simulink model "MX_Gateway"  (Simulink 9.0) (continued)
  CD_FAST_TRAVEL_THROTTLE_LIMIT = Bobcat.Parameter; CreateAliasCD( CD_FAST_TRAVEL_THROTTLE_LIMIT                        ,uint16(1000)               ,'ConstantDataPointer' ,'CONSTANT_DATA' ,'FAST_TRAVEL_THROTTLE_LIMIT')    [cdata.m]    (also in hc12_cdata.m; m4_cdata.m)
  CD_FLYWHEEL_TEETH = Bobcat.Parameter; CreateAliasCD( CD_FLYWHEEL_TEETH                                    ,uint8(83)                  ,'ConstantDataPointer' ,'CONSTANT_DATA' ,'FLYWHEEL_TEETH')    [cdata.m]    (also in hc12_cdata.m; m4_cdata.m)
  CD_FUEL_LEVEL_FILTER_FAST_VALUE = Bobcat.Parameter; CreateAliasCD( CD_FUEL_LEVEL_FILTER_FAST_VALUE                      ,uint8(5)                   ,'ConstantDataPointer' ,'CONSTANT_DATA' ,'FUEL_LEVEL_FILTER_FAST_VALUE')    [cdata.m]    (also in hc12_cdata.m; m4_cdata.m)
  CD_FUEL_LEVEL_FILTER_SLOW_VALUE = Bobcat.Parameter; CreateAliasCD( CD_FUEL_LEVEL_FILTER_SLOW_VALUE                      ,uint8(10)                  ,'ConstantDataPointer' ,'CONSTANT_DATA' ,'FUEL_LEVEL_FILTER_SLOW_VALUE')    [cdata.m]    (also in hc12_cdata.m; m4_cdata.m)
  CD_FUEL_PRIMARY_FEEDBACK_FUNCTION = Bobcat.Parameter; CreateAliasCD( CD_FUEL_PRIMARY_FEEDBACK_FUNCTION                    ,uint8(0)                   ,'ConstantDataPointer' ,'CONSTANT_DATA' ,'FUEL_PRIMARY_FEEDBACK_FUNCTION')    [cdata.m]    (also in hc12_cdata.m; m4_cdata.m)
  ... (+246 more)
undefined symbols (not defined in the file or in its folder): sigbuilder_block
undefined symbols (not defined in the file): CD_FUEL_RESOLUTION_TIMER
libraries: simulink x186, MX_Library x107
(part 10 of 48)

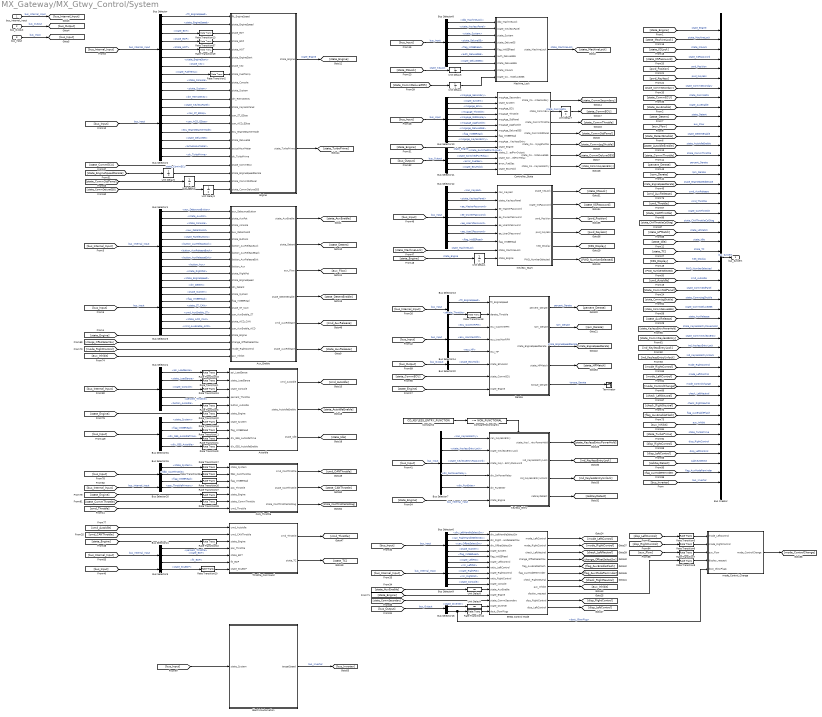
== system MX_Gateway/MX_Gtwy_Control/System ==
  bus_Internal_Input : Inport
  bus_Output : Inport  Port=2
  bus_Input : Inport  Port=3
  AutoIdle : Subsystem  RequirementInfo={} %GIDa_b4de8e37_b570_4228_8969_fb5e451b31f8  TreatAsAtomicUnit=on  SystemSampleTime=srate100  RTWFcnName=mod_engine_control  RTWFileName=mod_System
      settings: RTWSystemCode=Nonreusable function  RTWFileNameOpts=User specified
  Aux_Enable : Subsystem  TreatAsAtomicUnit=on  SystemSampleTime=srate  RTWFileName=mod_System
      settings: RTWSystemCode=Nonreusable function  RTWFcnNameOpts=Use subsystem name  RTWFileNameOpts=User specified
  Bus Creator : BusCreator  Inputs=48
  Bus Selector : BusSelector  OutputSignals=flt_EngineSpeed,state_EngineSpeed,state_ECT,state_EOP,state_HOT,state_EngineStart,state_Key,state_FuelTemp,state_Console
  Bus Selector1 : BusSelector  OutputSignals=aux_DebounceButton,state_AuxPot,state_Console,aux_DetentHold,state_PanelButtons,button_AuxReleaseAck,button_AuxReleaseBack,button_AuxReleaseExit,button_Aux,state_RightPot,state_EngineSpeed
  Bus Selector10 : BusSelector  OutputSignals=bus_CAN.bus_Secondary.msgAge_Secondary,bus_Controller.state_System,bus_CAN.bus_ECU.msgAge_ECU,bus_CAN.bus_Throttle.msgAge_Throttle,bus_CAN.bus_StdDisplay.msgAge_StdDisplay,bus_CAN.bus_JogShuttle.msgAge_JogShuttle,bus_CAN.bus_DeluxeG55.msgAge_DeluxeG55,bus_Controller.flag_InitEERead,bus_CAN.bus_KeylessEntry.msgAge_KeylessEntry
  Bus Selector11 : BusSelector  OutputSignals=state_SwitchedPwrOutput,state_SwitchedPwrRelay,error_FuelSec,state_ECUHold
  Bus Selector12 : BusSelector  OutputSignals=bus_CAN.bus_Keypad.can_Keypad,bus_CAN.bus_Keypad.state_KeylessPanel,bus_Controller.ee_MasterPassword,bus_Controller.ee_OwnerPassword,bus_Controller.ee_User1Password,bus_Controller.ee_User2Password,bus_Controller.flag_InitEERead
  Bus Selector13 : BusSelector  OutputSignals=flt_EngineSpeed,derate_Throttle
  Bus Selector14 : BusSelector  OutputSignals=bus_CAN.bus_ECU.ecu_isocMinRPM,bus_CAN.bus_ECU.ecu_isocMaxRPM,bus_CAN.bus_ECU.ecu_HP
  Bus Selector15 : BusSelector  OutputSignals=bus_Controller.state_System,bus_CAN.bus_Throttle.fdbk_CANThrottle,bus_Controller.flag_InitEERead
  Bus Selector16 : BusSelector  OutputSignals=state_Diverter,dout_GlowPlug
  Bus Selector2 : BusSelector  OutputSignals=bus_Controller.state_System,bus_DigitalInputs.din_RemoteKey,bus_CAN.bus_Keypad.state_KeylessPanel,bus_CAN.bus_ServiceTool.can_ST_EStop,bus_CAN.bus_ACD.can_ACD_EStop,bus_CAN.bus_ECU.ecu_EngineStarterMode,bus_CAN.bus_DeluxeG5.state_DeluxeG5,bus_Controller.actualHourMeter,bus_Controller.dv_TurboPrime
  Bus Selector20 : BusSelector  OutputSignals=pos_ThrottlePrimary
  Bus Selector21 : BusSelector  OutputSignals=percent_Throttle,state_EOT,flt_EOP
  Bus Selector22 : BusSelector  OutputSignals=state_ECUHold
  Bus Selector3 : BusSelector  OutputSignals=bus_DigitalInputs.din_Detent,bus_Controller.state_System,bus_Controller.flag_InitEERead,bus_CAN.bus_ServiceTool.state_ST_CAN,bus_CAN.bus_ServiceTool.cmd_AuxEnable_ST,bus_CAN.bus_ACD.state_ACD_CAN,bus_CAN.bus_ACD.cmd_AuxEnable_ACD
  Bus Selector31 : BusSelector  OutputSignals=bus_Controller.state_System,bus_Controller.flag_InitEERead,bus_CAN.bus_DeluxeG55.dlx_G55_AutoIdleTime,bus_CAN.bus_DeluxeG55.dlx_G55_AutoIdle
  Bus Selector4 : BusSelector  OutputSignals=psi_LoadSense,state_LoadSense,state_Console,percent_Throttle,button_AutoIdle
  Bus Selector5 : BusSelector  OutputSignals=bus_CAN.bus_ECU.state_ecuEOP
  Bus Selector6 : BusSelector  OutputSignals=bus_DigitalInputs.din_LeftHandleSelectSw,bus_CAN.bus_Secondary.can_RightHandleSelectSw,bus_CAN.bus_Secondary.can_OffsetSelectSw,bus_Controller.state_System,bus_Controller.flag_InitEERead
  Bus Selector7 : BusSelector  OutputSignals=bus_CAN.bus_KeylessEntry.can_KeylessEntry,bus_Controller.state_KeylessEntryLock,bus_Controller.state_KeylessEntryPassword,bus_DigitalInputs.din_SwPowerRelay,bus_DigitalInputs.din_RunEnter
  Bus Selector8 : BusSelector  OutputSignals=bus_Controller.ddp_MachineLock,bus_CAN.bus_Keypad.state_KeylessPanel,bus_Controller.state_System,bus_CAN.bus_DeluxeG5.state_DeluxeG5,bus_Controller.flag_InitEERead,bus_CAN.bus_DeluxeG55.auth_DeluxeG55,bus_CAN.bus_DeluxeG55.state_DeluxeG55
  Bus Selector9 : BusSelector  OutputSignals=state_LeftPot,rm_LeftPot,state_RightPot,rm_RightPot,state_Console
  CAN_Throttle : Subsystem  TreatAsAtomicUnit=on  SystemSampleTime=srate100  RTWFileName=mod_System
      settings: RTWSystemCode=Nonreusable function  RTWFileNameOpts=User specified
  CompareToConstant1 : simulink/Logic and Bit Operations/Compare To Constant  relop=~=  const=NON_FUNCTIONAL  OutDataTypeStr=boolean  ZeroCross=off
  Constant2 : Constant  Value=CD_KEYLESS_ENTRY_FUNCTION
  Controller_State : Subsystem  TreatAsAtomicUnit=on  SystemSampleTime=srate  RTWFileName=mod_System
      settings: RTWSystemCode=Nonreusable function  RTWFileNameOpts=User specified
  Derate : Subsystem  TreatAsAtomicUnit=on  SystemSampleTime=srate  RTWFileName=mod_System
      settings: RTWSystemCode=Nonreusable function  RTWFileNameOpts=User specified
  EMEA Control Mode : Subsystem  TreatAsAtomicUnit=on  RTWFileName=mod_System
      settings: RTWSystemCode=Nonreusable function  RTWFileNameOpts=User specified
  ElectricAutomation : Subsystem  TreatAsAtomicUnit=on  SystemSampleTime=srate100  RTWFcnName=mod_engine_control  RTWFileName=mod_System
      settings: RTWSystemCode=Nonreusable function  RTWFileNameOpts=User specified
  Engine : Subsystem  TreatAsAtomicUnit=on  SystemSampleTime=srate  RTWFileName=mod_System
      settings: RTWSystemCode=Nonreusable function  RTWFcnNameOpts=Use subsystem name  RTWFileNameOpts=User specified
  Keyless_Entry : Subsystem  TreatAsAtomicUnit=on  SystemSampleTime=srate  RTWFileName=mod_System
      settings: RTWSystemCode=Nonreusable function  RTWFileNameOpts=User specified
  Keyless_Start : Subsystem  TreatAsAtomicUnit=on  SystemSampleTime=srate  RTWFileName=mod_System
      settings: RTWSystemCode=Nonreusable function  RTWFileNameOpts=User specified
  Machine_Lock : Subsystem  TreatAsAtomicUnit=on  SystemSampleTime=srate  RTWFileName=mod_System
      settings: RTWSystemCode=Nonreusable function  RTWFileNameOpts=User specified
  Rate Transition{1..4,9..13,17..20,22..28,57,64} (22 alike) : RateTransition  Integrity=off
  Rate Transition5 : RateTransition  Integrity=off  OutPortSampleTime=srate100
  Rate Transition6 : RateTransition  Integrity=off  OutPortSampleTime=srate100
  Rate Transition7 : RateTransition  Integrity=off  OutPortSampleTime=srate100
  Rate Transition8 : RateTransition  OutPortSampleTime=srate100
  Terminator : Terminator
  Throttle_Command : Subsystem  TreatAsAtomicUnit=on  RTWFileName=mod_System
      settings: RTWSystemCode=Nonreusable function  RTWFileNameOpts=User specified
  Unit Delay{1..9} (9 alike) : UnitDelay  SampleTime=-1
      settings: AttributesFormatString=<tsample=%<SampleTime>>\n<initial=%<X0>>
  mode_Control_Change : Subsystem
  bus_System : Outport
  wires:
  [state_Engine] -> Bus Creator/1  "state_Engine"
  Bus Selector/4 -> Rate Transition57/1  "<state_EOP>"
  Rate Transition57/1 -> Engine/4(state_EOP)
  Bus Selector/2 -> Engine/2(state_EngineSpeed)  "<state_EngineSpeed>"
  Engine/1(state_Engine) -> [state_Engine]  "state_Engine"
  Bus Selector/3 -> Rate Transition13/1  "<state_ECT>"
  Bus Selector/6 -> Engine/6(state_EngineStart)  "<state_EngineStart>"
  Bus Selector/7 -> Engine/7(state_Key)  "<state_Key>"
  Rate Transition13/1 -> Engine/3(state_ECT)
  Rate Transition19/1 -> Engine/5(state_HOT)
  bus_Internal_Input/1 -> [bus_Internal_Input]  "bus_Internal_Input"
  bus_Output/1 -> [bus_Output]  "bus_Output"
  bus_Input/1 -> [bus_Input]  "bus_Input"
  [bus_Internal_Input] -> Bus Selector/1  "bus_Internal_Input"
  [bus_Input] -> Bus Selector2/1  "bus_Input"
  Bus Selector/1 -> Engine/1(flt_EngineSpeed)  "<flt_EngineSpeed>"
  Bus Selector/5 -> Rate Transition19/1  "<state_HOT>"
  [bus_Input] -> Bus Selector8/1  "bus_Input"
  Bus Selector8/1 -> Machine_Lock/1(ddp_MachineLock)  "<ddp_MachineLock>"
  Machine_Lock/1(state_MachineLock) -> [state_MachineLock]  "state_MachineLock"
  [state_MachineLock] -> Bus Creator/2  "state_MachineLock"
  Bus Selector8/2 -> Machine_Lock/2(state_KeylessPanel)  "<state_KeylessPanel>"
  [state_KSLock] -> Bus Creator/3  "state_KSLock"
  [state_KSPassword] -> Bus Creator/4  "state_KSPassword"
  [pwd_Position] -> Bus Creator/5  "pwd_Position"
  [pwd_Keyless] -> Bus Creator/6  "pwd_Keyless"
  Bus Selector8/3 -> Machine_Lock/3(state_System)  "<state_System>"
  [state_KSLock] -> Unit Delay5/1  "state_KSLock"
  Unit Delay5/1 -> Machine_Lock/8(state_KSLock)
  Bus Selector8/4 -> Machine_Lock/4(state_DeluxeG5)  "<state_DeluxeG5>"
  Bus Selector8/5 -> Machine_Lock/5(flag_InitEERead)  "<flag_InitEERead>"
  Controller_State/1(state_CommSecondary) -> [state_CommSecondary]
  [bus_Input] -> Bus Selector10/1  "bus_Input"
  Bus Selector10/1 -> Controller_State/1(msgAge_Secondary)  "<msgAge_Secondary>"
  Bus Selector10/2 -> Controller_State/2(state_System)  "<state_System>"
  [state_Engine] -> Controller_State/10(state_Engine)  "state_Engine"
  [bus_Output] -> Bus Selector11/1  "bus_Output"
  Bus Selector11/2 -> Controller_State/12(state_SwitchedPwrRelay)  "<state_SwitchedPwrRelay>"
  [state_CommSecondary] -> Bus Creator/7  "state_CommSecondary"
  Bus Selector10/3 -> Controller_State/3(msgAge_ECU)  "<msgAge_ECU>"
  Controller_State/2(state_CommECU) -> Unit Delay7/1  "state_CommECU"
  [state_CommECU] -> Bus Creator/8  "state_CommECU"
  [state_CommECU] -> Engine/19(state_CommECU)  "state_CommECU"
  Unit Delay7/1 -> [state_CommECU]
  Bus Selector11/1 -> Controller_State/11(state_SwitchedPwrOutput)  "<state_SwitchedPwrOutput>"
  Bus Selector11/3 -> Controller_State/13(error_FuelSec)  "<error_FuelSec>"
  Bus Selector11/4 -> Controller_State/14(state_ECUHold)  "<state_ECUHold>"
  Bus Selector2/1 -> Engine/10(state_System)  "<state_System>"
  Bus Selector2/2 -> Engine/11(din_RemoteKey)  "<din_RemoteKey>"
  Bus Selector2/3 -> Engine/12(state_KeylessPanel)  "<state_KeylessPanel>"
  Bus Selector2/5 -> Engine/14(can_ACD_EStop)  "<can_ACD_EStop>"
  Bus Selector2/6 -> Engine/15(ecu_EngineStarterMode)  "<ecu_EngineStarterMode>"
  [state_AuxEnable] -> Bus Creator/9  "state_AuxEnable"
  Aux_Enable/1(state_AuxEnable) -> [state_AuxEnable]
  [bus_Internal_Input] -> Bus Selector1/1  "bus_Internal_Input"
  Bus Selector1/1 -> Aux_Enable/1(aux_DebounceButton)  "<aux_DebounceButton>"
  Bus Selector1/2 -> Aux_Enable/2(state_AuxPot)  "<state_AuxPot>"
  [state_Engine] -> Aux_Enable/19(state_Engine)
  [bus_Input] -> Bus Selector3/1  "bus_Input"
  Bus Selector3/1 -> Aux_Enable/12(din_Detent)  "<din_Detent>"
  Aux_Enable/2(state_Detent) -> [state_Detent]
  Aux_Enable/3(aux_Flow) -> [aux_Flow]
  Aux_Enable/4(state_DetentEnable) -> [state_DetentEnable]
  [state_Detent] -> Bus Creator/10  "state_Detent"
  [aux_Flow] -> Bus Creator/11  "aux_Flow"
  [state_DetentEnable] -> Bus Creator/12  "state_DetentEnable"
  [state_AutoIdleEnable] -> Bus Creator/13  "state_AutoIdleEnable"
  Bus Selector10/4 -> Controller_State/4(msgAge_Throttle)  "<msgAge_Throttle>"
  Controller_State/3(state_CommThrottle) -> [state_CommThrottle]
  [state_CommThrottle] -> Bus Creator/14  "state_CommThrottle"
  [percent_Derate] -> Bus Creator/15  "percent_Derate"
  [rpm_Derate] -> Bus Creator/16  "rpm_Derate"
  [state_EngineSpeedDerate] -> Bus Creator/17  "state_EngineSpeedDerate"
  Bus Selector1/3 -> Aux_Enable/3(state_console)  "<state_Console>"
  Bus Selector1/4 -> Aux_Enable/4(aux_DetentHold)  "<aux_DetentHold>"
  Bus Selector3/2 -> Aux_Enable/13(state_System)  "<state_System>"
  [state_EngineSpeedDerate] -> Unit Delay6/1
  Unit Delay6/1 -> Engine/20(state_EngineSpeedDerate)
  [bus_Internal_Input] -> Bus Selector13/1  "bus_Input"
  Bus Selector13/1 -> Derate/1(flt_EngineSpeed)  "<flt_EngineSpeed>"
  [bus_Input] -> Bus Selector14/1  "bus_Input"
  Derate/1(percent_Derate) -> [percent_Derate]  "percent_Derate"
  Derate/2(rpm_Derate) -> [rpm_Derate]  "rpm_Derate"
  Bus Selector14/1 -> Derate/3(ecu_isocMinRPM)  "<ecu_isocMinRPM>"
  Bus Selector14/2 -> Derate/4(ecu_isocMaxRPM)  "<ecu_isocMaxRPM>"
  Derate/3(state_EngineSpeedDerate) -> [state_EngineSpeedDerate]  "state_EngineSpeedDerate"
  Bus Selector13/2 -> Rate Transition18/1  "<derate_Throttle>"
  Rate Transition18/1 -> Derate/2(derate_Throttle)
  Bus Selector3/3 -> Aux_Enable/14(flag_InitEERead)  "<flag_InitEERead>"
  Bus Selector1/5 -> Aux_Enable/5(state_Buttons)  "<state_PanelButtons>"
  Bus Selector2/4 -> Engine/13(can_ST_EStop)  "<can_ST_EStop>"
  Bus Selector3/4 -> Aux_Enable/15(state_ST_CAN)  "<state_ST_CAN>"
  Bus Selector3/5 -> Aux_Enable/16(can_AuxEnable_ST)  "<cmd_AuxEnable_ST>"
  Bus Selector3/6 -> Aux_Enable/17(state_ACD_CAN)  "<state_ACD_CAN>"
  Bus Selector3/7 -> Aux_Enable/18(can_AuxEnable_ACD)  "<cmd_AuxEnable_ACD>"
  Aux_Enable/5(cmd_AuxRelease) -> [cmd_AuxRelease]
  [cmd_AuxRelease] -> Bus Creator/18  "cmd_AuxRelease"
  Bus Selector1/6 -> Aux_Enable/6(button_AuxReleaseAck)  "<button_AuxReleaseAck>"
  Bus Selector1/7 -> Aux_Enable/7(button_AuxReleaseBack)  "<button_AuxReleaseBack>"
  Bus Selector1/8 -> Aux_Enable/8(button_AuxReleaseExit)  "<button_AuxReleaseExit>"
  Rate Transition24/1 -> AutoIdle/3(state_Console)
  Rate Transition22/1 -> AutoIdle/6(state_Engine)
  Rate Transition23/1 -> AutoIdle/1(psi_LoadSense)
  [bus_Input] -> Bus Selector31/1
  Bus Selector31/1 -> Rate Transition64/1  "<state_System>"
  Rate Transition64/1 -> AutoIdle/7(state_System)
  [bus_Internal_Input] -> Bus Selector4/1
  Bus Selector4/1 -> Rate Transition23/1  "<psi_LoadSense>"
  Bus Selector4/3 -> Rate Transition24/1  "<state_Console>"
  Bus Selector4/4 -> AutoIdle/4(percent_Throttle)  "<percent_Throttle>"
  [state_Engine] -> Rate Transition22/1
  Bus Selector4/2 -> Rate Transition1/1  "<state_LoadSense>"
  Rate Transition1/1 -> AutoIdle/2(state_LoadSense)
  AutoIdle/1(cmd_AutoIdle) -> [cmd_AutoIdle]
  [cmd_Throttle] -> Bus Creator/19  "cmd_Throttle"
  AutoIdle/2(state_AutoIdleEnable) -> [state_AutoIdleEnable]
  [bus_Input] -> Bus Selector15/1
  Bus Selector15/1 -> Rate Transition17/1  "<state_System>"
  Bus Selector15/2 -> Rate Transition25/1  "<fdbk_CANThrottle>"
  Bus Selector15/3 -> Rate Transition26/1  "<flag_InitEERead>"
  [state_Engine] -> Rate Transition27/1
  [state_CommThrottle] -> Rate Transition28/1
  [bus_Internal_Input] -> Bus Selector20/1  "bus_Internal_Input"
  Bus Selector20/1 -> CAN_Throttle/4(pos_Throttle)  "<pos_ThrottlePrimary>"
  CAN_Throttle/1(cmd_CANThrottle) -> [cmd_CANThrottle]
  CAN_Throttle/2(state_CANThrottle) -> [state_CANThrottle]
  CAN_Throttle/3(state_CANThrottleCalDiag) -> [state_CANThrottleCalDiag]
  [state_CANThrottle] -> Bus Creator/20  "state_CANThrottle"
  [state_CANThrottleCalDiag] -> Bus Creator/21  "state_CANThrottleCalDiag"
  Rate Transition17/1 -> CAN_Throttle/1(state_System)
  Rate Transition25/1 -> CAN_Throttle/2(fdbk_CANThrottle)
  Rate Transition26/1 -> CAN_Throttle/3(flag_InitEERead)
  Rate Transition27/1 -> CAN_Throttle/5(state_Engine)
  Rate Transition28/1 -> CAN_Throttle/6(state_CommThrottle)
  Bus Creator/1 -> bus_System/1  "bus_System"
  [state_HPMatch] -> Bus Creator/22  "state_HPMatch"
  Derate/4(state_HPMatch) -> [state_HPMatch]
  [bus_Output] -> Bus Selector22/1  "bus_Output"
  Bus Selector14/3 -> Derate/5(ecu_HP)  "<ecu_HP>"
  Bus Selector22/1 -> Derate/6(state_ECUHold)  "<state_ECUHold>"
  [state_CommECU] -> Derate/7(state_CommECU)
  Bus Selector/8 -> Rate Transition2/1  "<state_FuelTemp>"
  AutoIdle/3(state_Idle) -> [state_Idle]
  [state_Idle] -> Bus Creator/23  "state_Idle"
  Rate Transition2/1 -> Engine/8(state_FuelTemp)
  Throttle_Command/1(cmd_Throttle) -> [cmd_Throttle]
  [cmd_AutoIdle] -> Throttle_Command/1(cmd_AutoIdle)
  [bus_Internal_Input] -> Bus Selector21/1  "bus_Internal_Input"
  Bus Selector21/1 -> Throttle_Command/4(pos_Throttle)  "<percent_Throttle>"
  [cmd_CANThrottle] -> Throttle_Command/2(cmd_CANThrottle)
  Throttle_Command/2(state_TC) -> [state_TC]
  Bus Selector21/2 -> Throttle_Command/5(state_EOT)  "<state_EOT>"
  [state_TC] -> Bus Creator/24  "state_TC"
  [state_Engine] -> Rate Transition10/1
  Bus Selector4/5 -> Rate Transition3/1  "<button_AutoIdle>"
  Rate Transition3/1 -> AutoIdle/5(button_AutoIdle)
  Rate Transition10/1 -> Throttle_Command/3(state_Engine)
  [cmd_Throttle] -> CAN_Throttle/7(cmd_Throttle)
  [bus_Input] -> Bus Selector5/1
  Bus Selector5/1 -> Rate Transition20/1  "<state_ecuEOP>"
  Bus Selector21/3 -> Throttle_Command/6(flt_EOP)  "<flt_EOP>"
  Rate Transition20/1 -> Throttle_Command/7(state_ecuEOP)
  Bus Selector1/9 -> Aux_Enable/9(button_Aux)  "<button_Aux>"
  Keyless_Start/4(pwd_Keyless) -> [pwd_Keyless]
  Keyless_Start/3(pwd_Position) -> [pwd_Position]
  Keyless_Start/2(state_KSPassword) -> [state_KSPassword]
  Keyless_Start/1(state_KSLock) -> [state_KSLock]
  [bus_Input] -> Bus Selector12/1  "bus_Input"
  Keyless_Start/5(KSS_Display) -> [KSS_Display]
  Keyless_Start/6(PWD_NumberSelected) -> [PWD_NumberSelected]
  Bus Selector12/1 -> Keyless_Start/1(can_Keypad)  "<can_Keypad>"
  Bus Selector12/2 -> Keyless_Start/2(state_KeylessPanel)  "<state_KeylessPanel>"
  Bus Selector12/5 -> Keyless_Start/5(ee_User1Password)  "<ee_User1Password>"
  Bus Selector12/6 -> Keyless_Start/6(ee_User2Password)  "<ee_User2Password>"
  [state_MachineLock] -> Keyless_Start/8(state_MachineLock)  "state_MachineLock"
  [state_Engine] -> Unit Delay1/1  "state_Engine"
  Unit Delay1/1 -> Keyless_Start/9(state_Engine)
  [KSS_Display] -> Bus Creator/25  "KSS_Display"
  [PWD_NumberSelected] -> Bus Creator/26  "PWD_NumberSelected"
  Bus Selector12/3 -> Keyless_Start/3(ee_MasterPassword)  "<ee_MasterPassword>"
  Bus Selector12/4 -> Keyless_Start/4(ee_OwnerPassword)  "<ee_OwnerPassword>"
  Rate Transition11/1 -> AutoIdle/8(flag_InitEERead)
  Bus Selector31/2 -> Rate Transition11/1  "<flag_InitEERead>"
  Bus Selector12/7 -> Keyless_Start/7(flag_InitEERead)  "<flag_InitEERead>"
  [state_Engine] -> Derate/8(state_Engine)
  Derate/5(torque_Derate) -> Terminator/1  "torque_Derate"
  [cmd_AutoIdle] -> Bus Creator/27  "cmd_AutoIdle"
  Bus Selector10/7 -> Controller_State/7(msgAge_DeluxeG55)  "<msgAge_DeluxeG55>"
  Bus Selector10/5 -> Controller_State/5(msgAge_StdPanel)  "<msgAge_StdDisplay>"
  Bus Selector10/6 -> Controller_State/6(msgAge_JogShuttle)  "<msgAge_JogShuttle>"
  Controller_State/4(state_CommStdPanel) -> [state_CommStdPanel]
  Controller_State/5(state_CommJogShuttle) -> [state_CommJogShuttle]
  Controller_State/6(state_CommDeluxeG55) -> [state_CommDeluxeG55]
  [state_CommDeluxeG55] -> Bus Creator/30  "state_CommDeluxeG55"
  [state_CommStdPanel] -> Bus Creator/28  "state_CommStdPanel"
  [state_CommJogShuttle] -> Bus Creator/29  "state_CommJogShuttle"
  Bus Selector2/7 -> Engine/16(state_DeluxeG5)  "<state_DeluxeG5>"
  [state_CommDeluxeG55] -> Unit Delay3/1
  [state_CommStdPanel] -> Unit Delay2/1
  Unit Delay2/1 -> Engine/21(state_CommStdPanel)
  Unit Delay3/1 -> Engine/22(state_CommDeluxeG55)
  Rate Transition4/1 -> AutoIdle/9(dlx_G55_AutoIdleTime)
  Bus Selector31/3 -> Rate Transition4/1  "<dlx_G55_AutoIdleTime>"
  Aux_Enable/6(state_AuxRelease) -> [state_AuxRelease]
  [state_AuxRelease] -> Bus Creator/31  "state_AuxRelease"
  Bus Selector10/8 -> Controller_State/8(flag_InitEERead)  "<flag_InitEERead>"
  Bus Selector/9 -> Engine/9(state_Console)  "<state_Console>"
  Bus Selector8/6 -> Machine_Lock/6(auth_DeluxeG55)  "<auth_DeluxeG55>"
  [state_CommDeluxeG55] -> Unit Delay4/1
  Unit Delay4/1 -> Machine_Lock/9(state_CommDeluxeG55)
  [bus_Input] -> Bus Selector7/1  "bus_Input"
  Bus Selector7/1 -> Keyless_Entry/1(can_KeylessEntry)  "<can_KeylessEntry>"
  Keyless_Entry/1(state_KeylessEntryPowerHold) -> [state_KeylessEntryPowerHold]
  [state_KeylessEntryPowerHold] -> Bus Creator/32  "state_KeylessEntryPowerHold"
  Bus Selector10/9 -> Controller_State/9(msgAge_KeylessEntry)  "<msgAge_KeylessEntry>"
  Controller_State/7(state_CommKeylessEntry) -> [state_CommKeylessEntry]
  [state_CommKeylessEntry] -> Bus Creator/33  "state_CommKeylessEntry"
  Constant2/1 -> CompareToConstant1/1
  CompareToConstant1/1 -> Keyless_Entry/Enable
  Keyless_Entry/2(ind_KeylessEntryLock) -> [ind_KeylessEntryLock]
  Keyless_Entry/3(ind_KeylessEntryUnlock) -> [ind_KeylessEntryUnlock]
  [ind_KeylessEntryLock] -> Bus Creator/34  "ind_KeylessEntryLock"
  [ind_KeylessEntryUnlock] -> Bus Creator/35  "ind_KeylessEntryUnlock"
  Bus Selector2/8 -> Engine/17(actualHourMeter)  "<actualHourMeter>"
  Bus Selector2/9 -> Engine/18(dv_TurboPrime)  "<dv_TurboPrime>"
  [bus_Input] -> Bus Selector6/1  "bus_Input"
  Bus Selector6/1 -> EMEA Control Mode/1(din_LeftHandleSelectSw)  "<din_LeftHandleSelectSw>"
  [bus_Internal_Input] -> Bus Selector9/1  "bus_Internal_Input"
  Bus Selector9/1 -> EMEA Control Mode/6(state_LeftControl)  "<state_LeftPot>"
  Bus Selector9/2 -> EMEA Control Mode/7(ratio_LeftControl)  "<rm_LeftPot>"
  Bus Selector9/3 -> EMEA Control Mode/8(state_RightControl)  "<state_RightPot>"
  Bus Selector9/4 -> EMEA Control Mode/9(ratio_RightControl)  "<rm_RightPot>"
  [state_AuxEnable] -> Unit Delay8/1
  [mode_RightControl] -> Bus Creator/36  "mode_RightControl"
  [mode_LeftControl] -> Bus Creator/37  "mode_LeftControl"
  [mode_ControlChange] -> Bus Creator/38  "mode_ControlChange"
  Bus Selector6/2 -> EMEA Control Mode/2(din_RightHandleSelectSw)  "<can_RightHandleSelectSw>"
  Bus Selector6/3 -> EMEA Control Mode/3(din_OffsetSelectSw)  "<can_OffsetSelectSw>"
  EMEA Control Mode/1(mode_LeftControl) -> [mode_LeftControl]
  EMEA Control Mode/2(mode_RightControl) -> [mode_RightControl]
  Bus Selector1/10 -> Aux_Enable/10(state_RightPot)  "<state_RightPot>"
  EMEA Control Mode/3(check_LeftNeutral) -> [check_LeftNeutral]
  [check_LeftNeutral] -> Bus Creator/39  "check_LeftNeutral"
  [aux_Flow] -> Rate Transition7/1
  EMEA Control Mode/4(change_OffsetSelectSw) -> [change_OffsetSelectSw]
  [change_OffsetSelectSw] -> Aux_Enable/20(change_OffsetSelectSw)
  [mode_RightControl] -> Aux_Enable/21(mode_RightControl)
  Bus Selector6/4 -> EMEA Control Mode/4(state_System)  "<state_System>"
  Bus Selector9/5 -> EMEA Control Mode/10(state_console)  "<state_Console>"
  [state_Engine] -> EMEA Control Mode/12(state_Engine)
  Unit Delay8/1 -> EMEA Control Mode/11(state_AuxEnable)
  [check_RightNeutral] -> Bus Creator/40  "check_RightNeutral"
  [flag_AuxEnableFlash] -> Bus Creator/41  "flag_AuxEnableFlash"
  EMEA Control Mode/5(flag_AuxEnableFlash) -> [flag_AuxEnableFlash]
  EMEA Control Mode/7(check_RightNeutral) -> [check_RightNeutral]
  EMEA Control Mode/8(aux_Inhibit) -> [aux_Inhibit]
  [aux_Inhibit] -> Aux_Enable/22(aux_Inhibit)
  [aux_Inhibit] -> Bus Creator/42  "aux_Inhibit"
  [state_CommSecondary] -> EMEA Control Mode/13(state_CommSecondary)
  [bus_Output] -> Bus Selector16/1  "bus_Output"
  Bus Selector16/1 -> Unit Delay9/1  "<state_Diverter>"
  Unit Delay9/1 -> EMEA Control Mode/14(state_Diverter)
  Rate Transition5/1 -> mode_Control_Change/1(mode_LeftControl)
  Rate Transition6/1 -> mode_Control_Change/2(mode_RightControl)
  Rate Transition7/1 -> mode_Control_Change/3(aux_Flow)
  mode_Control_Change/1(mode_ControlChange) -> [mode_ControlChange]
  EMEA Control Mode/9(display_request) -> Rate Transition8/1
  Rate Transition8/1 -> mode_Control_Change/4(display_request)
  Engine/2(state_TurboPrime) -> [state_TurboPrime]
  [state_TurboPrime] -> Bus Creator/43  "state_TurboPrime"
  Bus Selector8/7 -> Machine_Lock/7(state_DeluxeG55)  "<state_DeluxeG55>"
  Bus Selector1/11 -> Aux_Enable/11(state_EngineSpeed)  "<state_EngineSpeed>"
  Bus Selector6/5 -> EMEA Control Mode/5(flag_InitEERead)  "<flag_InitEERead>"
  Bus Selector7/2 -> Keyless_Entry/2(state_KeylessEntryLock)  "<state_KeylessEntryLock>"
  Bus Selector7/3 -> Keyless_Entry/3(state_KeylessEntryPassword)  "<state_KeylessEntryPassword>"
  EMEA Control Mode/11(disp_LeftControl) -> [disp_LeftControl]
  EMEA Control Mode/10(disp_RightControl) -> [disp_RightControl]
  [disp_RightControl] -> Bus Creator/44  "disp_RightControl"
  [disp_LeftControl] -> Bus Creator/45  "disp_LeftControl"
  Rate Transition9/1 -> AutoIdle/10(dlx_G55_AutoIdleEnable)
  Bus Selector31/4 -> Rate Transition9/1  "<dlx_G55_AutoIdle>"
  Bus Selector16/2 -> mode_Control_Change/5(dout_GlowPlugs), Rate Transition12/1  "<dout_GlowPlug>"
  Rate Transition12/1 -> EMEA Control Mode/15(dout_GlowPlugs)
  [disp_LeftControl] -> Rate Transition5/1
  [disp_RightControl] -> Rate Transition6/1
  Bus Selector7/4 -> Keyless_Entry/4(din_SwPowerRelay)  "<din_SwPowerRelay>"
  Bus Selector7/5 -> Keyless_Entry/5(din_RunEnter)  "<din_RunEnter>"
  [cabKeyDetect] -> Bus Creator/46  "cabKeyDetect"
  Keyless_Entry/4(cabKeyDetect) -> [cabKeyDetect]
  [state_Engine] -> Keyless_Entry/6(state_Engine)  "bus_Internal_Input"
  EMEA Control Mode/6(flag_AuxModeReminder) -> [flag_AuxModeReminder]
  [flag_AuxModeReminder] -> Bus Creator/47  "flag_AuxModeReminder"
  [bus_Input] -> ElectricAutomation/1(state_System)
  [bus_Inverter] -> Bus Creator/48  "bus_Inverter"
  ElectricAutomation/1(targetSpeed) -> [bus_Inverter]  "bus_Inverter"
